simulink model "CCMLin2Mgr"  (R2022b) (continued)
== system CCMLin2Mgr == (continued)
  IntPm25StsFrmClima_IntPm25StsFrmClima : Outport  Port=19  OutDataTypeStr=Enum: PmSnsrSts  PortDimensions=1
  IntPm25VluFrmClima_IntPm25VluFrmClima : Outport  Port=20  OutDataTypeStr=UInt10  PortDimensions=1
  LIN2ScheTableReq_Val : Outport  Port=21  OutDataTypeStr=UInt8  PortDimensions=1
  wires:
  RlyCmdCmft_RlyCmdCmft/1 -> Lin2Mgr_Runnable_sys/1(RlyCmdCmft_RlyCmdCmft_read)
  SysServCarCfgSts_SysServCarID/1 -> Lin2Mgr_Runnable_sys/2(SysServCarCfgSts_SysServCarID_read)
  SysServCarCfgSts_SysServCCPHEXSensorSts/1 -> Lin2Mgr_Runnable_sys/4(SysServCarCfgSts_SysServCCPHEXSensorSts_read)
  SysServCarCfgSts_SysServCCPLin2AFUSts/1 -> Lin2Mgr_Runnable_sys/5(SysServCarCfgSts_SysServCCPLin2AFUSts_read)
  SysServCarCfgSts_SysServCCPLin2AIUSts/1 -> Lin2Mgr_Runnable_sys/6(SysServCarCfgSts_SysServCCPLin2AIUSts_read)
  SysServCarCfgSts_SysServCCPLin2CDSSts/1 -> Lin2Mgr_Runnable_sys/7(SysServCarCfgSts_SysServCCPLin2CDSSts_read)
  SysServCarCfgSts_SysServCCPLin2PMSISts/1 -> Lin2Mgr_Runnable_sys/8(SysServCarCfgSts_SysServCCPLin2PMSISts_read)
  SysServCarCfgSts_SysServCCPLin3VGASts/1 -> Lin2Mgr_Runnable_sys/9(SysServCarCfgSts_SysServCCPLin3VGASts_read)
  SysServCarCfgSts_SysServDUAirVentSnrSts/1 -> Lin2Mgr_Runnable_sys/10(SysServCarCfgSts_SysServDUAirVentSnrSts_read)
  SysServCarCfgSts_SysServDUBlowerPwmSignSts/1 -> Lin2Mgr_Runnable_sys/11(SysServCarCfgSts_SysServDUBlowerPwmSignSts_read)
  SysServCarCfgSts_SysServDUBlowerPwmSignTypeSts/1 -> Lin2Mgr_Runnable_sys/12(SysServCarCfgSts_SysServDUBlowerPwmSignTypeSts_read)
  SysServCarCfgSts_SysServDUBlowerPwmSts/1 -> Lin2Mgr_Runnable_sys/13(SysServCarCfgSts_SysServDUBlowerPwmSts_read)
  SysServCarCfgSts_SysServDUDCDefrostSts/1 -> Lin2Mgr_Runnable_sys/14(SysServCarCfgSts_SysServDUDCDefrostSts_read)
  SysServCarCfgSts_SysServDUDCModeSts/1 -> Lin2Mgr_Runnable_sys/15(SysServCarCfgSts_SysServDUDCModeSts_read)
  SysServCarCfgSts_SysServDUDCRecSts/1 -> Lin2Mgr_Runnable_sys/16(SysServCarCfgSts_SysServDUDCRecSts_read)
  SysServCarCfgSts_SysServDUDCSModeSts/1 -> Lin2Mgr_Runnable_sys/17(SysServCarCfgSts_SysServDUDCSModeSts_read)
  SysServCarCfgSts_SysServDUDCSTLSts/1 -> Lin2Mgr_Runnable_sys/18(SysServCarCfgSts_SysServDUDCSTLSts_read)
  SysServCarCfgSts_SysServDUDCTLSts/1 -> Lin2Mgr_Runnable_sys/19(SysServCarCfgSts_SysServDUDCTLSts_read)
  SysServCarCfgSts_SysServDUDCTRSts/1 -> Lin2Mgr_Runnable_sys/20(SysServCarCfgSts_SysServDUDCTRSts_read)
  SysServCarCfgSts_SysServDUFLFootSts/1 -> Lin2Mgr_Runnable_sys/21(SysServCarCfgSts_SysServDUFLFootSts_read)
  SysServCarCfgSts_SysServDUFLVentSts/1 -> Lin2Mgr_Runnable_sys/22(SysServCarCfgSts_SysServDUFLVentSts_read)
  SysServCarCfgSts_SysServDUFRFootSts/1 -> Lin2Mgr_Runnable_sys/23(SysServCarCfgSts_SysServDUFRFootSts_read)
  SysServCarCfgSts_SysServDUFRVentSts/1 -> Lin2Mgr_Runnable_sys/24(SysServCarCfgSts_SysServDUFRVentSts_read)
  SysServCarCfgSts_SysServDUIncarMotorSts/1 -> Lin2Mgr_Runnable_sys/25(SysServCarCfgSts_SysServDUIncarMotorSts_read)
  SysServCarCfgSts_SysServDULin1HBMFSts/1 -> Lin2Mgr_Runnable_sys/26(SysServCarCfgSts_SysServDULin1HBMFSts_read)
  SysServCarCfgSts_SysServDULin4HFAFSts/1 -> Lin2Mgr_Runnable_sys/27(SysServCarCfgSts_SysServDULin4HFAFSts_read)
  SysServCarCfgSts_SysServDUSFLFootSts/1 -> Lin2Mgr_Runnable_sys/28(SysServCarCfgSts_SysServDUSFLFootSts_read)
  SysServCarCfgSts_SysServDUSFLVentSts/1 -> Lin2Mgr_Runnable_sys/29(SysServCarCfgSts_SysServDUSFLVentSts_read)
  SysServCarCfgSts_SysServDUSFRFootSts/1 -> Lin2Mgr_Runnable_sys/30(SysServCarCfgSts_SysServDUSFRFootSts_read)
  SysServCarCfgSts_SysServDUSFRVentSts/1 -> Lin2Mgr_Runnable_sys/31(SysServCarCfgSts_SysServDUSFRVentSts_read)
  SysServCarCfgSts_SysServDUSnsrForFCmptmtTSts/1 -> Lin2Mgr_Runnable_sys/32(SysServCarCfgSts_SysServDUSnsrForFCmptmtTSts_read)
  SysServCarCfgSts_SysServDUSnsrForRair/1 -> Lin2Mgr_Runnable_sys/33(SysServCarCfgSts_SysServDUSnsrForRair_read)
  SysServCarCfgSts_SysServDUSnsrForRCmptmtTSts/1 -> Lin2Mgr_Runnable_sys/34(SysServCarCfgSts_SysServDUSnsrForRCmptmtTSts_read)
  AACAirClngErrSts_AACAirClngErrSts/1 -> Lin2Mgr_Runnable_sys/35(AACAirClngErrSts_AACAirClngErrSts_read)
  AFUGenErr_AFUGenErr/1 -> Lin2Mgr_Runnable_sys/36(AFUGenErr_AFUGenErr_read)
  AirClngErrSts_AirClngErrSts/1 -> Lin2Mgr_Runnable_sys/40(AirClngErrSts_AirClngErrSts_read)
  AirFragFanErr_AirFragFanErr/1 -> Lin2Mgr_Runnable_sys/41(AirFragFanErr_AirFragFanErr_read)
  AirFragMot1Err_AirFragMot1Err/1 -> Lin2Mgr_Runnable_sys/42(AirFragMot1Err_AirFragMot1Err_read)
  AirFragMot2Err_AirFragMot2Err/1 -> Lin2Mgr_Runnable_sys/43(AirFragMot2Err_AirFragMot2Err_read)
  AirFragMot3Err_AirFragMot3Err/1 -> Lin2Mgr_Runnable_sys/44(AirFragMot3Err_AirFragMot3Err_read)
  AirFragMot4Err_AirFragMot4Err/1 -> Lin2Mgr_Runnable_sys/45(AirFragMot4Err_AirFragMot4Err_read)
  AirFragMot5Err_AirFragMot5Err/1 -> Lin2Mgr_Runnable_sys/46(AirFragMot5Err_AirFragMot5Err_read)
  AirFragOvrVoltErr_AirFragOvrVoltErr/1 -> Lin2Mgr_Runnable_sys/47(AirFragOvrVoltErr_AirFragOvrVoltErr_read)
  AirFragTempErr_AirFragTempErr/1 -> Lin2Mgr_Runnable_sys/48(AirFragTempErr_AirFragTempErr_read)
  AirFragUndVoltErr_AirFragUndVoltErr/1 -> Lin2Mgr_Runnable_sys/49(AirFragUndVoltErr_AirFragUndVoltErr_read)
  AQSAirQuality_AQSAirQuality/1 -> Lin2Mgr_Runnable_sys/53(AQSAirQuality_AQSAirQuality_read)
  AQSCOxstatus_AQSCOxstatus/1 -> Lin2Mgr_Runnable_sys/54(AQSCOxstatus_AQSCOxstatus_read)
  AQSNH3status_AQSNH3status/1 -> Lin2Mgr_Runnable_sys/55(AQSNH3status_AQSNH3status_read)
  AQSNoxStatus_AQSNoxStatus/1 -> Lin2Mgr_Runnable_sys/56(AQSNoxStatus_AQSNoxStatus_read)
  CarCfgForClimaCtrl_CarCfgForAirClng/1 -> Lin2Mgr_Runnable_sys/57(CarCfgForClimaCtrl_CarCfgForAirClng_read)
  CarCfgForClimaCtrl_CarCfgForAirClnrAdv/1 -> Lin2Mgr_Runnable_sys/58(CarCfgForClimaCtrl_CarCfgForAirClnrAdv_read)
  CarCfgForClimaCtrl_CarCfgForAirFrag/1 -> Lin2Mgr_Runnable_sys/59(CarCfgForClimaCtrl_CarCfgForAirFrag_read)
  CarCfgForClimaCtrl_CarCfgForAirMtrlSnsr/1 -> Lin2Mgr_Runnable_sys/60(CarCfgForClimaCtrl_CarCfgForAirMtrlSnsr_read)
  CarCfgForClimaCtrl_CarCfgForAirQly/1 -> Lin2Mgr_Runnable_sys/61(CarCfgForClimaCtrl_CarCfgForAirQly_read)
  CarCfgForClimaCtrl_CarCfgForAmntOfSeat/1 -> Lin2Mgr_Runnable_sys/62(CarCfgForClimaCtrl_CarCfgForAmntOfSeat_read)
  CarCfgForClimaCtrl_CarCfgForAutDemst/1 -> Lin2Mgr_Runnable_sys/63(CarCfgForClimaCtrl_CarCfgForAutDemst_read)
  CarCfgForClimaCtrl_CarCfgForAuxWtrPmp/1 -> Lin2Mgr_Runnable_sys/64(CarCfgForClimaCtrl_CarCfgForAuxWtrPmp_read)
  CarCfgForClimaCtrl_CarCfgForBRAND/1 -> Lin2Mgr_Runnable_sys/65(CarCfgForClimaCtrl_CarCfgForBRAND_read)
  CarCfgForClimaCtrl_CarCfgForBypVlvInHvac/1 -> Lin2Mgr_Runnable_sys/66(CarCfgForClimaCtrl_CarCfgForBypVlvInHvac_read)
  CarCfgForClimaCtrl_CarCfgForCabinOxgenSupply/1 -> Lin2Mgr_Runnable_sys/67(CarCfgForClimaCtrl_CarCfgForCabinOxgenSupply_read)
  CarCfgForClimaCtrl_CarCfgForClsVlvInHvac/1 -> Lin2Mgr_Runnable_sys/68(CarCfgForClimaCtrl_CarCfgForClsVlvInHvac_read)
  CarCfgForClimaCtrl_CarCfgForCmprAcTyp/1 -> Lin2Mgr_Runnable_sys/69(CarCfgForClimaCtrl_CarCfgForCmprAcTyp_read)
  CarCfgForClimaCtrl_CarCfgForCmptmtClng/1 -> Lin2Mgr_Runnable_sys/70(CarCfgForClimaCtrl_CarCfgForCmptmtClng_read)
  CarCfgForClimaCtrl_CarCfgForElectricLiftingModule/1 -> Lin2Mgr_Runnable_sys/71(CarCfgForClimaCtrl_CarCfgForElectricLiftingModule_read)
  CarCfgForClimaCtrl_CarCfgForElecVentAdjmt/1 -> Lin2Mgr_Runnable_sys/72(CarCfgForClimaCtrl_CarCfgForElecVentAdjmt_read)
  CarCfgForClimaCtrl_CarCfgForExtVentnClimaCtrl/1 -> Lin2Mgr_Runnable_sys/73(CarCfgForClimaCtrl_CarCfgForExtVentnClimaCtrl_read)
  CarCfgForClimaCtrl_CarCfgForFlcTyp/1 -> Lin2Mgr_Runnable_sys/74(CarCfgForClimaCtrl_CarCfgForFlcTyp_read)
  CarCfgForClimaCtrl_CarCfgForFoldCSD/1 -> Lin2Mgr_Runnable_sys/75(CarCfgForClimaCtrl_CarCfgForFoldCSD_read)
  CarCfgForClimaCtrl_CarCfgForFuTyp/1 -> Lin2Mgr_Runnable_sys/76(CarCfgForClimaCtrl_CarCfgForFuTyp_read)
  CarCfgForClimaCtrl_CarCfgForGlassTyp/1 -> Lin2Mgr_Runnable_sys/77(CarCfgForClimaCtrl_CarCfgForGlassTyp_read)
  CarCfgForClimaCtrl_CarCfgForHeadRadar/1 -> Lin2Mgr_Runnable_sys/78(CarCfgForClimaCtrl_CarCfgForHeadRadar_read)
  CarCfgForClimaCtrl_CarCfgForHeatPmp/1 -> Lin2Mgr_Runnable_sys/79(CarCfgForClimaCtrl_CarCfgForHeatPmp_read)
  CarCfgForClimaCtrl_CarCfgForHeatrDurgDrvg/1 -> Lin2Mgr_Runnable_sys/80(CarCfgForClimaCtrl_CarCfgForHeatrDurgDrvg_read)
  CarCfgForClimaCtrl_CarCfgForHeatrTyp/1 -> Lin2Mgr_Runnable_sys/81(CarCfgForClimaCtrl_CarCfgForHeatrTyp_read)
  CarCfgForClimaCtrl_CarCfgForHvacFilTyp/1 -> Lin2Mgr_Runnable_sys/82(CarCfgForClimaCtrl_CarCfgForHvacFilTyp_read)
  CarCfgForClimaCtrl_CarCfgForHvacFlrSnsrForT/1 -> Lin2Mgr_Runnable_sys/83(CarCfgForClimaCtrl_CarCfgForHvacFlrSnsrForT_read)
  CarCfgForClimaCtrl_CarCfgForHvacHeatrSnsrT/1 -> Lin2Mgr_Runnable_sys/84(CarCfgForClimaCtrl_CarCfgForHvacHeatrSnsrT_read)
  CarCfgForClimaCtrl_CarCfgForHvacRe/1 -> Lin2Mgr_Runnable_sys/85(CarCfgForClimaCtrl_CarCfgForHvacRe_read)
  CarCfgForClimaCtrl_CarCfgForHvacTyp/1 -> Lin2Mgr_Runnable_sys/86(CarCfgForClimaCtrl_CarCfgForHvacTyp_read)
  CarCfgForClimaCtrl_CarCfgForInteriorViewCamera/1 -> Lin2Mgr_Runnable_sys/87(CarCfgForClimaCtrl_CarCfgForInteriorViewCamera_read)
  CarCfgForClimaCtrl_CarCfgForLifeDetection/1 -> Lin2Mgr_Runnable_sys/88(CarCfgForClimaCtrl_CarCfgForLifeDetection_read)
  CarCfgForClimaCtrl_CarCfgForLoVoltElecCfg/1 -> Lin2Mgr_Runnable_sys/89(CarCfgForClimaCtrl_CarCfgForLoVoltElecCfg_read)
  CarCfgForClimaCtrl_CarCfgForNavSysandIntnmap/1 -> Lin2Mgr_Runnable_sys/90(CarCfgForClimaCtrl_CarCfgForNavSysandIntnmap_read)
  CarCfgForClimaCtrl_CarCfgForNrOfDoor/1 -> Lin2Mgr_Runnable_sys/91(CarCfgForClimaCtrl_CarCfgForNrOfDoor_read)
  CarCfgForClimaCtrl_CarCfgForPipeInFuTank/1 -> Lin2Mgr_Runnable_sys/92(CarCfgForClimaCtrl_CarCfgForPipeInFuTank_read)
  CarCfgForClimaCtrl_CarCfgForPowerSeats/1 -> Lin2Mgr_Runnable_sys/93(CarCfgForClimaCtrl_CarCfgForPowerSeats_read)
  CarCfgForClimaCtrl_CarCfgForPreClng/1 -> Lin2Mgr_Runnable_sys/94(CarCfgForClimaCtrl_CarCfgForPreClng_read)
  CarCfgForClimaCtrl_CarCfgForPrkgCDSNotAvl/1 -> Lin2Mgr_Runnable_sys/95(CarCfgForClimaCtrl_CarCfgForPrkgCDSNotAvl_read)
  CarCfgForClimaCtrl_CarCfgForPrkgClima/1 -> Lin2Mgr_Runnable_sys/96(CarCfgForClimaCtrl_CarCfgForPrkgClima_read)
  CarCfgForClimaCtrl_CarCfgForPrpsnTyp/1 -> Lin2Mgr_Runnable_sys/97(CarCfgForClimaCtrl_CarCfgForPrpsnTyp_read)
  CarCfgForClimaCtrl_CarCfgForPTCInFrontHVAC/1 -> Lin2Mgr_Runnable_sys/98(CarCfgForClimaCtrl_CarCfgForPTCInFrontHVAC_read)
  CarCfgForClimaCtrl_CarCfgForRemEngStrtPreCdn/1 -> Lin2Mgr_Runnable_sys/99(CarCfgForClimaCtrl_CarCfgForRemEngStrtPreCdn_read)
  CarCfgForClimaCtrl_CarCfgForRoofHatch/1 -> Lin2Mgr_Runnable_sys/100(CarCfgForClimaCtrl_CarCfgForRoofHatch_read)
  CarCfgForClimaCtrl_CarCfgForSeatHeatgCal/1 -> Lin2Mgr_Runnable_sys/101(CarCfgForClimaCtrl_CarCfgForSeatHeatgCal_read)
  CarCfgForClimaCtrl_CarCfgForSeatHeatgForRowFirst/1 -> Lin2Mgr_Runnable_sys/102(CarCfgForClimaCtrl_CarCfgForSeatHeatgForRowFirst_read)
  CarCfgForClimaCtrl_CarCfgForSeatHeatgForRowSec/1 -> Lin2Mgr_Runnable_sys/103(CarCfgForClimaCtrl_CarCfgForSeatHeatgForRowSec_read)
  CarCfgForClimaCtrl_CarCfgForSeatVentnForRowFirst/1 -> Lin2Mgr_Runnable_sys/104(CarCfgForClimaCtrl_CarCfgForSeatVentnForRowFirst_read)
  CarCfgForClimaCtrl_CarCfgForSeatVentnForRowSec/1 -> Lin2Mgr_Runnable_sys/105(CarCfgForClimaCtrl_CarCfgForSeatVentnForRowSec_read)
  CarCfgForClimaCtrl_CarCfgForSecRowVentnCenter/1 -> Lin2Mgr_Runnable_sys/106(CarCfgForClimaCtrl_CarCfgForSecRowVentnCenter_read)
  CarCfgForClimaCtrl_CarCfgForSecRowVentnSide/1 -> Lin2Mgr_Runnable_sys/107(CarCfgForClimaCtrl_CarCfgForSecRowVentnSide_read)
  CarCfgForClimaCtrl_CarCfgForSmallFlowCyc/1 -> Lin2Mgr_Runnable_sys/108(CarCfgForClimaCtrl_CarCfgForSmallFlowCyc_read)
  CarCfgForClimaCtrl_CarCfgForSmkSnsr/1 -> Lin2Mgr_Runnable_sys/109(CarCfgForClimaCtrl_CarCfgForSmkSnsr_read)
  CarCfgForClimaCtrl_CarCfgForSnsrForCmptmtT/1 -> Lin2Mgr_Runnable_sys/110(CarCfgForClimaCtrl_CarCfgForSnsrForCmptmtT_read)
  CarCfgForClimaCtrl_CarCfgForSnsrHumPrsnt/1 -> Lin2Mgr_Runnable_sys/111(CarCfgForClimaCtrl_CarCfgForSnsrHumPrsnt_read)
  CarCfgForClimaCtrl_CarCfgForStartDevice/1 -> Lin2Mgr_Runnable_sys/112(CarCfgForClimaCtrl_CarCfgForStartDevice_read)
  CarCfgForClimaCtrl_CarCfgForSteerWhlHeatg/1 -> Lin2Mgr_Runnable_sys/113(CarCfgForClimaCtrl_CarCfgForSteerWhlHeatg_read)
  CarCfgForClimaCtrl_CarCfgForSteerWhlPosn/1 -> Lin2Mgr_Runnable_sys/114(CarCfgForClimaCtrl_CarCfgForSteerWhlPosn_read)
  CarCfgForClimaCtrl_CarCfgForSunSnsr/1 -> Lin2Mgr_Runnable_sys/115(CarCfgForClimaCtrl_CarCfgForSunSnsr_read)
  CarCfgForClimaCtrl_CarCfgForTelmNodTyp/1 -> Lin2Mgr_Runnable_sys/116(CarCfgForClimaCtrl_CarCfgForTelmNodTyp_read)
  CarCfgForClimaCtrl_CarCfgForThermMngt/1 -> Lin2Mgr_Runnable_sys/117(CarCfgForClimaCtrl_CarCfgForThermMngt_read)
  CarCfgForClimaCtrl_CarCfgForVehTyp/1 -> Lin2Mgr_Runnable_sys/118(CarCfgForClimaCtrl_CarCfgForVehTyp_read)
  CarCfgForClimaCtrl_CarCfgForVentLiftMotorExiSts/1 -> Lin2Mgr_Runnable_sys/119(CarCfgForClimaCtrl_CarCfgForVentLiftMotorExiSts_read)
  CarCfgForClimaCtrl_CarCfgForVgaMotorExistSts/1 -> Lin2Mgr_Runnable_sys/120(CarCfgForClimaCtrl_CarCfgForVgaMotorExistSts_read)
  CarCfgForClimaCtrl_CarCfgForWadingAssistSystem/1 -> Lin2Mgr_Runnable_sys/121(CarCfgForClimaCtrl_CarCfgForWadingAssistSystem_read)
  CarCfgForClimaCtrl_CarCfgForWindHeatd/1 -> Lin2Mgr_Runnable_sys/122(CarCfgForClimaCtrl_CarCfgForWindHeatd_read)
  CarCfgForClimaCtrl_CarCfgWindFollowHumanMov/1 -> Lin2Mgr_Runnable_sys/123(CarCfgForClimaCtrl_CarCfgWindFollowHumanMov_read)
  ClimRlyCmd_ClimRlyCmd/1 -> Lin2Mgr_Runnable_sys/127(ClimRlyCmd_ClimRlyCmd_read)
  CmpmtInAirPmAQI_CmpmtInAirPmAQI/1 -> Lin2Mgr_Runnable_sys/128(CmpmtInAirPmAQI_CmpmtInAirPmAQI_read)
  CmpmtInAirPmDnsty_CmpmtInAirPmDnsty/1 -> Lin2Mgr_Runnable_sys/129(CmpmtInAirPmDnsty_CmpmtInAirPmDnsty_read)
  CmptmtOxygenConcQFNgcc_CmptmtOxygenConcQFNgcc/1 -> Lin2Mgr_Runnable_sys/130(CmptmtOxygenConcQFNgcc_CmptmtOxygenConcQFNgcc_read)
  CmptmtOxygenConcVluNgcc_CmptmtOxygenConcVluNgcc/1 -> Lin2Mgr_Runnable_sys/131(CmptmtOxygenConcVluNgcc_CmptmtOxygenConcVluNgcc_read)
  HvacCfgForClimaCtrl_HvacCfgForHvacFrontBlowerType/1 -> Lin2Mgr_Runnable_sys/133(HvacCfgForClimaCtrl_HvacCfgForHvacFrontBlowerType_read)
  HvacCfgForClimaCtrl_HvacCfgForHvacLowSideRETXVESts/1 -> Lin2Mgr_Runnable_sys/134(HvacCfgForClimaCtrl_HvacCfgForHvacLowSideRETXVESts_read)
  HvacCfgForClimaCtrl_HvacCfgForHvacMiscExistSts/1 -> Lin2Mgr_Runnable_sys/135(HvacCfgForClimaCtrl_HvacCfgForHvacMiscExistSts_read)
  HvacCfgForClimaCtrl_HvacCfgForHvacMotorExistSts/1 -> Lin2Mgr_Runnable_sys/136(HvacCfgForClimaCtrl_HvacCfgForHvacMotorExistSts_read)
  HvacCfgForClimaCtrl_HvacCfgForHvacReBlowerType/1 -> Lin2Mgr_Runnable_sys/137(HvacCfgForClimaCtrl_HvacCfgForHvacReBlowerType_read)
  HvacCfgForClimaCtrl_HvacCfgForHvacReVentPstn/1 -> Lin2Mgr_Runnable_sys/138(HvacCfgForClimaCtrl_HvacCfgForHvacReVentPstn_read)
  HvacCfgForClimaCtrl_HvacCfgForHvacSensorExistSts/1 -> Lin2Mgr_Runnable_sys/139(HvacCfgForClimaCtrl_HvacCfgForHvacSensorExistSts_read)
  HvacCfgForClimaCtrl_HvacCfgForHvacSnsrRCmptmtESts/1 -> Lin2Mgr_Runnable_sys/140(HvacCfgForClimaCtrl_HvacCfgForHvacSnsrRCmptmtESts_read)
  HvacCfgForClimaCtrl_HvacCfgForHvacSnsrReHexESts/1 -> Lin2Mgr_Runnable_sys/141(HvacCfgForClimaCtrl_HvacCfgForHvacSnsrReHexESts_read)
  HvacFanReqRe_HvacFanReqRe/1 -> Lin2Mgr_Runnable_sys/142(HvacFanReqRe_HvacFanReqRe_read)
  HvacFanSts2HvacFanBattU2_HvacFanSts2HvacFanBattU2/1 -> Lin2Mgr_Runnable_sys/143(HvacFanSts2HvacFanBattU2_HvacFanSts2HvacFanBattU2_read)
  HvacFanSts2HvacFanExt2_HvacFanSts2HvacFanExt2/1 -> Lin2Mgr_Runnable_sys/144(HvacFanSts2HvacFanExt2_HvacFanSts2HvacFanExt2_read)
  HvacFanSts2HvacFanHw2_HvacFanSts2HvacFanHw2/1 -> Lin2Mgr_Runnable_sys/145(HvacFanSts2HvacFanHw2_HvacFanSts2HvacFanHw2_read)
  HvacFanSts2HvacFanMotU2_HvacFanSts2HvacFanMotU2/1 -> Lin2Mgr_Runnable_sys/146(HvacFanSts2HvacFanMotU2_HvacFanSts2HvacFanMotU2_read)
  HvacFanSts2HvacFanReSts1_HvacFanSts2HvacFanReSts1/1 -> Lin2Mgr_Runnable_sys/147(HvacFanSts2HvacFanReSts1_HvacFanSts2HvacFanReSts1_read)
  HvacFanSts2HvacFanReSts2_HvacFanSts2HvacFanReSts2/1 -> Lin2Mgr_Runnable_sys/148(HvacFanSts2HvacFanReSts2_HvacFanSts2HvacFanReSts2_read)
  HvacFanSts2HvacFanSw2_HvacFanSts2HvacFanSw2/1 -> Lin2Mgr_Runnable_sys/149(HvacFanSts2HvacFanSw2_HvacFanSts2HvacFanSw2_read)
  HvacFanSts2HvacFanT2_HvacFanSts2HvacFanT2/1 -> Lin2Mgr_Runnable_sys/150(HvacFanSts2HvacFanT2_HvacFanSts2HvacFanT2_read)
  HvacFanVoltReqRe_HvacFanVoltReqRe/1 -> Lin2Mgr_Runnable_sys/151(HvacFanVoltReqRe_HvacFanVoltReqRe_read)
  InPm25FanErr_InPm25FanErr/1 -> Lin2Mgr_Runnable_sys/152(InPm25FanErr_InPm25FanErr_read)
  InPm25SensrIntErr_InPm25SensrIntErr/1 -> Lin2Mgr_Runnable_sys/153(InPm25SensrIntErr_InPm25SensrIntErr_read)
  InPm25SnrTempErr_InPm25SnrTempErr/1 -> Lin2Mgr_Runnable_sys/154(InPm25SnrTempErr_InPm25SnrTempErr_read)
  InPm25SnrVoltgRangErr_InPm25SnrVoltgRangErr/1 -> Lin2Mgr_Runnable_sys/155(InPm25SnrVoltgRangErr_InPm25SnrVoltgRangErr_read)
  InPm25SnsrElecErr_InPm25SnsrElecErr/1 -> Lin2Mgr_Runnable_sys/156(InPm25SnsrElecErr_InPm25SnsrElecErr_read)
  InPm25SnsrSts_InPm25SnsrSts/1 -> Lin2Mgr_Runnable_sys/157(InPm25SnsrSts_InPm25SnsrSts_read)
  IntCo2CircElecErr_IntCo2CircElecErr/1 -> Lin2Mgr_Runnable_sys/158(IntCo2CircElecErr_IntCo2CircElecErr_read)
  IntCo2HvErr_IntCo2HvErr/1 -> Lin2Mgr_Runnable_sys/159(IntCo2HvErr_IntCo2HvErr_read)
  IntCo2LvErr_IntCo2LvErr/1 -> Lin2Mgr_Runnable_sys/160(IntCo2LvErr_IntCo2LvErr_read)
  OxygenConcSnsrElecErr_OxygenConcSnsrElecErr/1 -> Lin2Mgr_Runnable_sys/162(OxygenConcSnsrElecErr_OxygenConcSnsrElecErr_read)
  OxygenConcSnsrElecErrNgcc_OxygenConcSnsrElecErrNgcc/1 -> Lin2Mgr_Runnable_sys/163(OxygenConcSnsrElecErrNgcc_OxygenConcSnsrElecErrNgcc_read)
  OxygenConcSnsrIntErr_OxygenConcSnsrIntErr/1 -> Lin2Mgr_Runnable_sys/164(OxygenConcSnsrIntErr_OxygenConcSnsrIntErr_read)
  OxygenConcSnsrIntErrNgcc_OxygenConcSnsrIntErrNgcc/1 -> Lin2Mgr_Runnable_sys/165(OxygenConcSnsrIntErrNgcc_OxygenConcSnsrIntErrNgcc_read)
  OxygenConcSnsrOvrVoltRangErr_OxygenConcSnsrOvrVoltRangErr/1 -> Lin2Mgr_Runnable_sys/166(OxygenConcSnsrOvrVoltRangErr_OxygenConcSnsrOvrVoltRangErr_read)
  OxygenConcSnsrStsNgcc_OxygenConcSnsrStsNgcc/1 -> Lin2Mgr_Runnable_sys/167(OxygenConcSnsrStsNgcc_OxygenConcSnsrStsNgcc_read)
  OxygenConcSnsrTempErr_OxygenConcSnsrTempErr/1 -> Lin2Mgr_Runnable_sys/168(OxygenConcSnsrTempErr_OxygenConcSnsrTempErr_read)
  OxygenConcSnsrTempErrNgcc_OxygenConcSnsrTempErrNgcc/1 -> Lin2Mgr_Runnable_sys/169(OxygenConcSnsrTempErrNgcc_OxygenConcSnsrTempErrNgcc_read)
  OxygenConcSnsrUndVoltRangErr_OxygenConcSnsrUndVoltRangErr/1 -> Lin2Mgr_Runnable_sys/170(OxygenConcSnsrUndVoltRangErr_OxygenConcSnsrUndVoltRangErr_read)
  OxygenConcSnsrUndVoltRangErrNgcc_OxygenConcSnsrUndVoltRangErrNgcc/1 -> Lin2Mgr_Runnable_sys/171(OxygenConcSnsrUndVoltRangErrNgcc_OxygenConcSnsrUndVoltRangErrNgcc_read)
  PwrSplyToSnsrForAirQly_PwrSplyToSnsrForAirQly/1 -> Lin2Mgr_Runnable_sys/174(PwrSplyToSnsrForAirQly_PwrSplyToSnsrForAirQly_read)
  RHFAScheTableReq_Val/1 -> Lin2Mgr_Runnable_sys/175(RHFAScheTableReq_Val_read)
  SysServCarCfgSts_SysServCCPBGMNum/1 -> Lin2Mgr_Runnable_sys/177(SysServCarCfgSts_SysServCCPBGMNum_read)
  SysServCarCfgSts_SysServCCPLin2AQSSts/1 -> Lin2Mgr_Runnable_sys/178(SysServCarCfgSts_SysServCCPLin2AQSSts_read)
  SysServCarCfgSts_SysServDUDCFLMdeSts/1 -> Lin2Mgr_Runnable_sys/179(SysServCarCfgSts_SysServDUDCFLMdeSts_read)
  SysServCarCfgSts_SysServDUReBlowerPwmSignSts/1 -> Lin2Mgr_Runnable_sys/180(SysServCarCfgSts_SysServDUReBlowerPwmSignSts_read)
  SysServCarCfgSts_SysServDUReBlowerPwmSts/1 -> Lin2Mgr_Runnable_sys/181(SysServCarCfgSts_SysServDUReBlowerPwmSts_read)
  SysServCarCfgSts_SysServFrntHEXSnsrPosn/1 -> Lin2Mgr_Runnable_sys/182(SysServCarCfgSts_SysServFrntHEXSnsrPosn_read)
  SysServCarCfgSts_SysServLowSideRETXVSts/1 -> Lin2Mgr_Runnable_sys/183(SysServCarCfgSts_SysServLowSideRETXVSts_read)
  SysServCarCfgSts_SysServReHEXSnsrPosn/1 -> Lin2Mgr_Runnable_sys/184(SysServCarCfgSts_SysServReHEXSnsrPosn_read)
  SysServCarCfgSts_SysServRHFASts/1 -> Lin2Mgr_Runnable_sys/185(SysServCarCfgSts_SysServRHFASts_read)
  SysServCarCfgSts_SysServSnsrForFCmptmtTType/1 -> Lin2Mgr_Runnable_sys/186(SysServCarCfgSts_SysServSnsrForFCmptmtTType_read)
  Lin2Mgr_Runnable_sys/1(AirQlyLin_AirQlyLin_write) -> AirQlyLin_AirQlyLin/1
  Lin2Mgr_Runnable_sys/2(HvacFanSet2HvacFanRxFrq2_HvacFanSet2HvacFanRxFrq2_write) -> HvacFanSet2HvacFanRxFrq2_HvacFanSet2HvacFanRxFrq2/1
  Lin2Mgr_Runnable_sys/3(Lin2ActvReady_Lin2ActvReady_write) -> Lin2ActvReady_Lin2ActvReady/1
  Lin2Mgr_Runnable_sys/4(Lin2MgrDtcServ2_AACGenElectrFail_write) -> Lin2MgrDtcServ2_AACGenElectrFail/1
  Lin2Mgr_Runnable_sys/5(Lin2MgrDtcServ2_IOSMGenElectrFail_write) -> Lin2MgrDtcServ2_IOSMGenElectrFail/1
  Lin2Mgr_Runnable_sys/6(Lin2MgrDtcServ2_IOSMInterFail_write) -> Lin2MgrDtcServ2_IOSMInterFail/1
  Lin2Mgr_Runnable_sys/7(ReVbmrSts_ReVbmrSts_write) -> ReVbmrSts_ReVbmrSts/1
  Lin2Mgr_Runnable_sys/8(AirFragErrSts_AirFragErrSts_write) -> AirFragErrSts_AirFragErrSts/1
  Lin2Mgr_Runnable_sys/9(AirPmQf_AirPmQf_write) -> AirPmQf_AirPmQf/1
  Lin2Mgr_Runnable_sys/10(HvacFanSet2HvacFanIMaxMax2_HvacFanSet2HvacFanIMaxMax2_write) -> HvacFanSet2HvacFanIMaxMax2_HvacFanSet2HvacFanIMaxMax2/1
  Lin2Mgr_Runnable_sys/11(HvacFanSet2HvacFanIMaxMin2_HvacFanSet2HvacFanIMaxMin2_write) -> HvacFanSet2HvacFanIMaxMin2_HvacFanSet2HvacFanIMaxMin2/1
  Lin2Mgr_Runnable_sys/12(HvacFanSet2HvacFanKneeU2_HvacFanSet2HvacFanKneeU2_write) -> HvacFanSet2HvacFanKneeU2_HvacFanSet2HvacFanKneeU2/1
  Lin2Mgr_Runnable_sys/13(HvacFanSet2HvacFanRamp2_HvacFanSet2HvacFanRamp2_write) -> HvacFanSet2HvacFanRamp2_HvacFanSet2HvacFanRamp2/1
  Lin2Mgr_Runnable_sys/14(HvacFanSet2HvacFanSeln2_HvacFanSet2HvacFanSeln2_write) -> HvacFanSet2HvacFanSeln2_HvacFanSet2HvacFanSeln2/1
  Lin2Mgr_Runnable_sys/15(HvacFanSet2HvacFanSftyRunThd2_HvacFanSet2HvacFanSftyRunThd2_write) -> HvacFanSet2HvacFanSftyRunThd2_HvacFanSet2HvacFanSftyRunThd2/1
  Lin2Mgr_Runnable_sys/16(HvacFanSet2HvacFanSpd2_HvacFanSet2HvacFanSpd2_write) -> HvacFanSet2HvacFanSpd2_HvacFanSet2HvacFanSpd2/1
  Lin2Mgr_Runnable_sys/17(HvacFanSet2HvacSftyRunSpd2_HvacFanSet2HvacSftyRunSpd2_write) -> HvacFanSet2HvacSftyRunSpd2_HvacFanSet2HvacSftyRunSpd2/1
  Lin2Mgr_Runnable_sys/18(IntPm25LvlFrmClima_IntPm25LvlFrmClima_write) -> IntPm25LvlFrmClima_IntPm25LvlFrmClima/1
  Lin2Mgr_Runnable_sys/19(IntPm25StsFrmClima_IntPm25StsFrmClima_write) -> IntPm25StsFrmClima_IntPm25StsFrmClima/1
  Lin2Mgr_Runnable_sys/20(IntPm25VluFrmClima_IntPm25VluFrmClima_write) -> IntPm25VluFrmClima_IntPm25VluFrmClima/1
  Lin2Mgr_Runnable_sys/21(LIN2ScheTableReq_Val_write) -> LIN2ScheTableReq_Val/1
  BswLifeCycle_LifeCycle/1 -> Lin2Mgr_Runnable_sys/187(BswLifeCycle_LifeCycle_read)
  SerNoPMSI_SerNoPMSI/1 -> [SerNoPMSI_SerNoPMSI_Tag]
  [SerNoPMSI_SerNoPMSI_Tag] -> Lin2Mgr_Runnable_sys/176(SerNoPMSI_SerNoPMSI_read)
  [SerNoPMSI_SerNoPMSI_Tag] -> Lin2Mgr_SerNoPMSI_sys/1(SerNoPMSI_SerNoPMSI_read)
  PMSIPartNoHw_PMSIPartNoHw/1 -> [PMSIPartNoHw_PMSIPartNoHw_Tag]
  [PMSIPartNoHw_PMSIPartNoHw_Tag] -> Lin2Mgr_PMSIPartNoHw_sys/1(PMSIPartNoHw_PMSIPartNoHw_read)
  [PMSIPartNoHw_PMSIPartNoHw_Tag] -> Lin2Mgr_Runnable_sys/173(PMSIPartNoHw_PMSIPartNoHw_read)
  PMSIPartNo10Hw_PMSIPartNo10Hw/1 -> [PMSIPartNo10Hw_PMSIPartNo10Hw_Tag]
  [PMSIPartNo10Hw_PMSIPartNo10Hw_Tag] -> Lin2Mgr_PMSIPartNo10Hw_sys/1(PMSIPartNo10Hw_PMSIPartNo10Hw_read)
  [PMSIPartNo10Hw_PMSIPartNo10Hw_Tag] -> Lin2Mgr_Runnable_sys/172(PMSIPartNo10Hw_PMSIPartNo10Hw_read)
  KeepComActvL2_KeepComActvL2/1 -> [KeepComActvL2_KeepComActvL2_Tag]
  [KeepComActvL2_KeepComActvL2_Tag] -> Lin2Mgr_KeepComActv_sys/1(KeepComActvL2_KeepComActvL2_read)
  [KeepComActvL2_KeepComActvL2_Tag] -> Lin2Mgr_Runnable_sys/161(KeepComActvL2_KeepComActvL2_read)
  CDSSerNo_CDSSerNo/1 -> [CDSSerNo_CDSSerNo_Tag]
  [CDSSerNo_CDSSerNo_Tag] -> Lin2Mgr_CDSSerNo_sys/1(CDSSerNo_CDSSerNo_read)
  [CDSSerNo_CDSSerNo_Tag] -> Lin2Mgr_Runnable_sys/126(CDSSerNo_CDSSerNo_read)
  CDSPartNoCmpl_CDSPartNoCmpl/1 -> [CDSPartNoCmpl_CDSPartNoCmpl_Tag]
  [CDSPartNoCmpl_CDSPartNoCmpl_Tag] -> Lin2Mgr_CDSPartNoCmpl_sys/1(CDSPartNoCmpl_CDSPartNoCmpl_read)
  [CDSPartNoCmpl_CDSPartNoCmpl_Tag] -> Lin2Mgr_Runnable_sys/125(CDSPartNoCmpl_CDSPartNoCmpl_read)
  CDSPartNo10Cmpl_CDSPartNo10Cmpl/1 -> [CDSPartNo10Cmpl_CDSPartNo10Cmpl_Tag]
  [CDSPartNo10Cmpl_CDSPartNo10Cmpl_Tag] -> Lin2Mgr_CDSPartNo10Cmpl_sys/1(CDSPartNo10Cmpl_CDSPartNo10Cmpl_read)
  [CDSPartNo10Cmpl_CDSPartNo10Cmpl_Tag] -> Lin2Mgr_Runnable_sys/124(CDSPartNo10Cmpl_CDSPartNo10Cmpl_read)
  AIUSerNo_AIUSerNo/1 -> [AIUSerNo_AIUSerNo_Tag]
  [AIUSerNo_AIUSerNo_Tag] -> Lin2Mgr_AIUSerNo_sys/1(AIUSerNo_AIUSerNo_read)
  [AIUSerNo_AIUSerNo_Tag] -> Lin2Mgr_Runnable_sys/52(AIUSerNo_AIUSerNo_read)
  AIUPartNoCmpl_AIUPartNoCmpl/1 -> [AIUPartNoCmpl_AIUPartNoCmpl_Tag]
  [AIUPartNoCmpl_AIUPartNoCmpl_Tag] -> Lin2Mgr_AIUPartNoCmpl_sys/1(AIUPartNoCmpl_AIUPartNoCmpl_read)
  [AIUPartNoCmpl_AIUPartNoCmpl_Tag] -> Lin2Mgr_Runnable_sys/51(AIUPartNoCmpl_AIUPartNoCmpl_read)
  AIUPartNo10Cmpl_AIUPartNo10Cmpl/1 -> [AIUPartNo10Cmpl_AIUPartNo10Cmpl_Tag]
  [AIUPartNo10Cmpl_AIUPartNo10Cmpl_Tag] -> Lin2Mgr_AIUPartNo10Cmpl_sys/1(AIUPartNo10Cmpl_AIUPartNo10Cmpl_read)
  [AIUPartNo10Cmpl_AIUPartNo10Cmpl_Tag] -> Lin2Mgr_Runnable_sys/50(AIUPartNo10Cmpl_AIUPartNo10Cmpl_read)
  AFUSerNo_AFUSerNo/1 -> [AFUSerNo_AFUSerNo_Tag]
  [AFUSerNo_AFUSerNo_Tag] -> Lin2Mgr_AFUSerNo_sys/1(AFUSerNo_AFUSerNo_read)
  [AFUSerNo_AFUSerNo_Tag] -> Lin2Mgr_Runnable_sys/39(AFUSerNo_AFUSerNo_read)
  AFUPartNoCmpl_AFUPartNoCmpl/1 -> [AFUPartNoCmpl_AFUPartNoCmpl_Tag]
  [AFUPartNoCmpl_AFUPartNoCmpl_Tag] -> Lin2Mgr_AFUPartNoCmpl_sys/1(AFUPartNoCmpl_AFUPartNoCmpl_read)
  [AFUPartNoCmpl_AFUPartNoCmpl_Tag] -> Lin2Mgr_Runnable_sys/38(AFUPartNoCmpl_AFUPartNoCmpl_read)
  SysServCarCfgSts_SysServCCPAQSSts/1 -> [SysServCarCfgSts_SysServCCPAQSSts_Tag]
  [SysServCarCfgSts_SysServCCPAQSSts_Tag] -> Lin2Mgr_AFUPartNo10Cmpl_sys/3(SysServCarCfgSts_SysServCCPAQSSts_read)
  [SysServCarCfgSts_SysServCCPAQSSts_Tag] -> Lin2Mgr_Runnable_sys/3(SysServCarCfgSts_SysServCCPAQSSts_read)
  HvacCfgForClimaCtrl_HvacCfgForAQSTypes/1 -> [HvacCfgForClimaCtrl_HvacCfgForAQSTypes_Tag]
  [HvacCfgForClimaCtrl_HvacCfgForAQSTypes_Tag] -> Lin2Mgr_AFUPartNo10Cmpl_sys/2(HvacCfgForClimaCtrl_HvacCfgForAQSTypes_read)
  [HvacCfgForClimaCtrl_HvacCfgForAQSTypes_Tag] -> Lin2Mgr_Runnable_sys/132(HvacCfgForClimaCtrl_HvacCfgForAQSTypes_read)
  AFUPartNo10Cmpl_AFUPartNo10Cmpl/1 -> [AFUPartNo10Cmpl_AFUPartNo10Cmpl_Tag]
  [AFUPartNo10Cmpl_AFUPartNo10Cmpl_Tag] -> Lin2Mgr_AFUPartNo10Cmpl_sys/1(AFUPartNo10Cmpl_AFUPartNo10Cmpl_read)
  [AFUPartNo10Cmpl_AFUPartNo10Cmpl_Tag] -> Lin2Mgr_Runnable_sys/37(AFUPartNo10Cmpl_AFUPartNo10Cmpl_read)
  Lin2Mgr_AFUPartNo10Cmpl/1 -> Lin2Mgr_AFUPartNo10Cmpl_sys/Trigger
  Lin2Mgr_AFUPartNoCmpl/1 -> Lin2Mgr_AFUPartNoCmpl_sys/Trigger
  Lin2Mgr_AFUSerNo/1 -> Lin2Mgr_AFUSerNo_sys/Trigger
  Lin2Mgr_AIUPartNo10Cmpl/1 -> Lin2Mgr_AIUPartNo10Cmpl_sys/Trigger
  Lin2Mgr_AIUPartNoCmpl/1 -> Lin2Mgr_AIUPartNoCmpl_sys/Trigger
  Lin2Mgr_AIUSerNo/1 -> Lin2Mgr_AIUSerNo_sys/Trigger
  Lin2Mgr_CDSPartNo10Cmpl/1 -> Lin2Mgr_CDSPartNo10Cmpl_sys/Trigger
  Lin2Mgr_CDSPartNoCmpl/1 -> Lin2Mgr_CDSPartNoCmpl_sys/Trigger
  Lin2Mgr_CDSSerNo/1 -> Lin2Mgr_CDSSerNo_sys/Trigger
  Lin2Mgr_KeepComActv/1 -> Lin2Mgr_KeepComActv_sys/Trigger
  Lin2Mgr_PMSIPartNo10Hw/1 -> Lin2Mgr_PMSIPartNo10Hw_sys/Trigger
  Lin2Mgr_PMSIPartNoHw/1 -> Lin2Mgr_PMSIPartNoHw_sys/Trigger
  Lin2Mgr_Runnable/1 -> Lin2Mgr_Runnable_sys/Trigger
  Lin2Mgr_SerNoPMSI/1 -> Lin2Mgr_SerNoPMSI_sys/Trigger
